simulink model "ofdm_txrx_mimo"  (Simulink 7.1)
settings: Solver=ode45  StartTime=0.0  StopTime=6e3  RelTol=1e-3
PreLoadFcn:
  | ofdm_rx_mimo_init
InitFcn:
  | ofdm_rx_mimo_init
StartFcn:
  | ofdm_rx_mimo_init
values from neighbouring files (symbols this model uses):
  BER_RAM_init_size = 2048    [ofdm_tx_mimo_init.m]
  CPLength = 16    [ofdm_tx_mimo_init.m]
  CRCPolynomial = hex2dec('04c11db7')    [ofdm_tx_mimo_init.m]
  freqOffset_track_filtCoef_i = hex2dec('2500')/2^32    [ofdm_rx_mimo_init.m]
  freqOffset_track_filtCoef_p = hex2dec('d0000')/2^32    [ofdm_rx_mimo_init.m]
  hi = zeros(1,length(cci))    [ofdm_rx_mimo_init.m]
  hr = zeros(1,length(ccr))    [ofdm_rx_mimo_init.m]
  max_CP_length = 16    [ofdm_tx_mimo_init.m]
  max_num_baseRateSymbols = 31    [ofdm_tx_mimo_init.m]
  max_num_subcarriers = 64    [ofdm_tx_mimo_init.m]
  max_num_trainingSymbols = 15    [ofdm_tx_mimo_init.m]
  max_numBytes = 16384    [ofdm_tx_mimo_init.m]
  max_OFDM_symbols = 2047    [ofdm_tx_mimo_init.m]
  modConstellation_qam16 = .75*[1 3 -1 -3]./3    [ofdm_tx_mimo_init.m]
  modConstellation_qam256 = 0.9375*[3 1 5 7 11 9 13 15 -3 -1 -5 -7 -11 -9 -13 -15]./15    [ofdm_tx_mimo_init.m]
  modConstellation_qam64 = 0.875*[3 1 5 7 -3 -1 -5 -7]./7    [ofdm_tx_mimo_init.m]
  modConstellation_qpsk = [1 -1]./sqrt(2)    [ofdm_tx_mimo_init.m]
  numBaseRateSymbols = 2    [ofdm_tx_mimo_init.m]
  numSubcarriers = 64    [ofdm_tx_mimo_init.m]
  numTrainingSymbols = 2    [ofdm_tx_mimo_init.m]
  pilot1_indicies = 7 + ( (64-7) * 2^16)    (= 3735559)    [ofdm_tx_mimo_init.m]
  pilot2_indicies = 21 + ( (64-21) * 2^16)    (= 2818069)    [ofdm_tx_mimo_init.m]
  pilotValue_neg = hex2dec('8000') + (2^16 * 0)    [ofdm_tx_mimo_init.m]
  pilotValue_pos = hex2dec('7FFF') + (2^16 * 0)    [ofdm_tx_mimo_init.m]
  pkt_crossCorr_thresh = 0.7    [ofdm_rx_mimo_init.m]
  pkt_energy_thresh = 0    [ofdm_rx_mimo_init.m]
  pkt_numPayloadBytes = 1400+42-6    (= 1436)    [ofdm_tx_mimo_init.m]
  pktByteNums = numHeaderBytes +  				byteIndex_numPayloadBytes(1)*2^8 +  				byteIndex_numPayloadBytes(2)*2^16 +  				byteIndex_simpleDynModMasks * 2^24    [ofdm_rx_mimo_init.m]
  PktDet_Delay = 26 + Symbol_Timing_Offset*2^7    (= 1050)    [ofdm_rx_mimo_init.m]
  preamble = 6*[0 shortsyms_10 longsyms_2]    [ofdm_tx_mimo_init.m]
  preamble_I = real(preamble)    [ofdm_tx_mimo_init.m]
  preamble_Q = imag(preamble)    [ofdm_tx_mimo_init.m]
  RAM_init_size = max_numBytes    (= 16384)    [ofdm_tx_mimo_init.m]
  reg_Interrupt_PktBuf_Control = reg_InterruptControl +  	1 * 2^16 +  	0 * 2^24    (= 65656)    [ofdm_rx_mimo_init.m]
  rx_controlBits = 1 * 2  		 + 1 * 4  		 + 0 * 8  		 + 1 * 16 * rx_SISO_Mode  		 + 1 * 32  		 + 1 * 64  		 + 0 * 128  		 + 1 * 256  		 + 0 * 512  		 + 1 * 1024  		 + 0 * 2048  		 + 1 * 4096  		 + 1 * 8192  		 + 0 * 16384  		 + 1 * 65536  		 + 1 * 131072  		 + 0 * 262144  		 + 1 * 524288    [ofdm_rx_mimo_init.m]
  Rx_PhaseNoiseTrack_K = 0.6094    [ofdm_rx_mimo_init.m]
  rx_postEq_scaling = round(rxScaling*2^11) * (1 + 2^16)    [ofdm_rx_mimo_init.m]
  RxDataScrambling_Seq = [40 198 78 63 82 173 102 245 48 111 172 115 147 230 216 93 72 65 62 2 205 242 122 90 128 83 105 97 73 10 5 252]    [ofdm_tx_mimo_init.m]
  simOnly_numSamples = length(preamble)+( (numSubcarriers+CPLength)*(numTrainingSymbols + numBaseRateSymbols + numFullRateSymbols/2) )    [ofdm_tx_mimo_init.m]
  train = [train train]    [ofdm_tx_mimo_init.m]
  tx_controlBits = (antB_preambleShift * 2^4) + (2^0 * tx_SISO_Mode) + 4*0 + 8*1    (= 73)    [ofdm_tx_mimo_init.m]
  TxDataScrambling_Seq = [40 198 78 63 82 173 102 245 48 111 172 115 147 230 216 93 72 65 62 2 205 242 122 90 128 83 105 97 73 10 5 252]    [ofdm_tx_mimo_init.m]
  txReg_symbolCounts = (2^16 * numFullRateSymbols) + (2^8 *numBaseRateSymbols ) + numTrainingSymbols    [ofdm_tx_mimo_init.m]
  TxRx_FFTScaling = bin2dec('010111') + (bin2dec('000101') * 2^6)    [ofdm_tx_mimo_init.m]
undefined symbols (not defined in the file or in its folder): AntA, AntB, BaseRate, CRC_table_gen, gcbh, intfilt, pktTiming_count_payloadStart, pktTiming_symbol_length, xlBlockLoadCallback, xlGetSimulinkPeriod
libraries: xbsIndex_r4 x4212, dspmlti4 x4, dspsigops x4, commchan3 x2, simulink x2, commrflib2 x2, dspsrcs4 x1
(part 1 of 43)
settings Model: Creator=Administrator  LastModifiedBy=Administrator

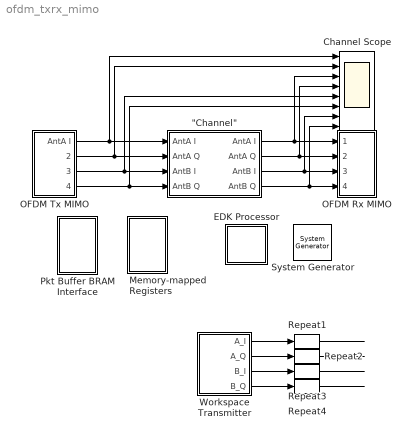
== system ofdm_txrx_mimo ==
  " System Generator" : xbsIndex_r4/ System Generator  Tag=genX  SystemSampleTime=-1  xilinxfamily=virtex2p  part=xc2vp70  speed=-6  package=ff1517  synthesis_tool=XST  clock_wrapper=Clock Enables  directory=./OFDM_txrx_v03_newerTxScaling  testbench=off  simulink_period=1  sysclk_period=10  dcm_input_clock_period=100  incr_netlist=off  trim_vbits=Everywhere in SubSystem  dbl_ovrd=According to Block Masks  core_generation=According to Block Masks  run_coregen=off  deprecated_control=off  eval_field=0  block_type=sysgen
      settings: block_version=8.2.02
  "\"Channel\"" : Subsystem  mask{h=[1 0; 0 1] * 0.3, freqOffset=1e-4, awgnSNR=500, phNoise=-5000}
      mask prompts: h "2x2 Channel Matrix"; freqOffset "Freq Offset (phase inc [0,1])"; awgnSNR "SNR (dB)"; phNoise "Phase Noise"
      mask help: This block implements a simple static 2x2 channel. The one parameter sets the 2x2 channel matrix. h=[1 0;0 1] would be perfectly orthogonal channels. The norm of the matrix should be 1 to avoid overflow.
  Channel Scope : Scope  NumInputPorts=8
      settings: TimeRange=3000  YMin=-1~-1~-0.3~-0.3~-1~-1~-1~-1  YMax=0.75~0.75~0.2~0.2~1~1~1~1  SaveName=ScopeData49
  EDK Processor : Subsystem  LoadFcn=xlBlockLoadCallback(gcbh);  mask(Xilinx EDK Processor Block){mode=EDK pcore generation, xmp=, MemVisToProc=<qt bgcolor="#FFFFFF"><div><img src="C:\Xilinx\10.1\DSP_Tools\common\bin\..\..\sysgen\data\images\registerplus.gif"> &lt;&lt;Rx_BER_Errors&gt;&gt;<br></div><div><img src="C:\Xilinx\10.1\DSP_Tools\common\bin\..\..\sysgen\data\images\registerplus.gif"> &lt;&lt;Rx_BER_TotalBits&gt;&gt;<br></div><div><img src="C:\Xilinx\10.1\DSP_Tools\common\bin\..\..\sysgen\data\images\registerplus.gif"> &lt;&lt;Rx_Constellation_Scaling_w&gt;&gt;<br></div><div><img src="C:\Xilinx\10.1\DSP_Tools\common\bin\..\..\sysgen\data\images\registerplus.gif"> &lt;&lt;Rx_ControlBits_r&gt;&gt;<br></div><div><img src="C:\Xilinx\10.1\DSP_Tools\common\bin\..\..\sysgen\data\images\registerplus.gif"> &lt;&lt;Rx_ControlBits_w&gt;&gt;<br></div><div><img src="C:\Xilinx\10.1\DSP_Tools\common\bin\..\..\sysgen\data\images\registerplus.gif"> &lt;&lt;Rx_EVM_w&gt;&gt;<br></div><div><img src="C:\Xilinx\10.1\DSP_Tools\common\bin\..\..\sysgen\data\images\registerplus.gif"> &lt;&lt;Rx_FreqOffFilt_B_KI_w&gt;&gt;<br></div><div><img src="C:\Xilinx\10.1\DSP_Tools\common\bin\..\..\sysgen\data\images\registerplus.gif"> &lt;&lt;Rx_FreqOffFilt_B_KP_w&gt;&gt;<br></div><div><img src="C:\Xilinx\10.1\DSP_Tools\common\bin\..\..\sysgen\data\images\registerplus.gif"> &lt;&lt;Rx_Gains&gt;&gt;<br></div><div><img src="C:\Xilinx\10.1\DSP_Tools\common\bin\..\..\sysgen\data\images\registerplus.gif"> &lt;&lt;Rx_OFDM_SymbolCounts_r&gt;&gt;<br></div><div><img src="C:\Xilinx\10.1\DSP_Tools\common\bin\..\..\sysgen\data\images\registerplus.gif"> &lt;&lt;Rx_OFDM_SymbolCounts_w&gt;&gt;<br></div><div><img src="C:\Xilinx\10.1\DSP_Tools\common\bin\..\..\sysgen\data\images\registerplus.gif"> &lt;&lt;Rx_PhaseNoiseTrack_K_w&gt;&gt;<br></div><div><img src="C:\Xilinx\10.1\DSP_Tools\common\bin\..\..\sysgen\data\images\registerplus.gif"> &lt;&lt;Rx_PktDet_Delay_r&gt;&gt;<br></div><div><img src="C:\Xilinx\10.1\DSP_Tools\common\bin\..\..\sysgen\data\images\registerplus.gif"> &lt;&lt;Rx_PktDet_Delay_w&gt;&gt;<br></div><div><img src="C:\Xilinx\10.1\DSP_Tools\common\bin\..\..\sysgen\data\images\registerplus.gif"> &lt;&lt;Rx_PktDet_LongCorr_Params_r&gt;&gt;<br></div><div><img src="C:\Xilinx\10.1\DSP_Tools\common\bin\..\..\sysgen\data\images\registerplus.gif"> &lt;&lt;Rx_PktDet_LongCorr_Params_w&gt;&gt;<br></div><div><img src="C:\Xilinx\10.1\DSP_Tools\common\bin\..\..\sysgen\data\images\registerplus.gif"> &lt;&lt;Rx_collision&gt;&gt;<br></div><div><img src="C:\Xilinx\10.1\DSP_Tools\common\bin\..\..\sysgen\data\images\registerplus.gif"> &lt;&lt;Rx_pktByteNums_r&gt;&gt;<br></div><div><img src="C:\Xilinx\10.1\DSP_Tools\common\bin\..\..\sysgen\data\images\registerplus.gif"> &lt;&lt;Rx_pktByteNums_w&gt;&gt;<br></div><div><img src="C:\Xilinx\10.1\DSP_Tools\common\bin\..\..\sysgen\data\images\registerplus.gif"> &lt;&lt;Rx_pktDet_Tresholds_w&gt;&gt;<br></div><div><img src="C:\Xilinx\10.1\DSP_Tools\common\bin\..\..\sysgen\data\images\registerplus.gif"> &lt;&lt;Rx_pktDone_interruptStatus&gt;&gt;<br></div><div><img src="C:\Xilinx\10.1\DSP_Tools\common\bin\..\..\sysgen\data\images\registerplus.gif"> &lt;&lt;TxRx_FFT_Scaling_w&gt;&gt;<br></div><div><img src="C:\Xilinx\10.1\DSP_Tools\common\bin\..\..\sysgen\data\images\registerplus.gif"> &lt;&lt;TxRx_Interrupt_PktBuf_Ctrl_r&gt;&gt;<br></div><div><img src="C:\Xilinx\10.1\DSP_Tools\common\bin\..\..\sysgen\data\images\registerplus.gif"> &lt;&lt;TxRx_Interrupt_PktBuf_Ctrl_w&gt;&gt;<br></div><div><img src="C:\Xilinx\10.1\DSP_Tools\common\bin\..\..\sysgen\data\images\registerplus.gif"> &lt;&lt;Tx_ControlBits_r&gt;&gt;<br></div><div><img src="C:\Xilinx\10.1\DSP_Tools\common\bin\..\..\sysgen\data\images\registerplus.gif"> &lt;&lt;Tx_ControlBits_w&gt;&gt;<br></div><div><img src="C:\Xilinx\10.1\DSP_Tools\common\bin\..\..\sysgen\data\images\registerplus.gif"> &lt;&lt;Tx_OFDM_SymCounts_r&gt;&gt;<br></div><div><img src="C:\Xilinx\10.1\DSP_Tools\common\bin\..\..\sysgen\data\images\registerplus.gif"> &lt;&lt;Tx_OFDM_SymCounts_w&gt;&gt;<br></div><div><img src="C:\Xilinx\10.1\DSP_Tools\common\bin\..\..\sysgen\data\images\registerplus.gif"> &lt;&lt;Tx_Pilots_Index1_w&gt;&gt;<br></div><div><img src="C:\Xilinx\10.1\DSP_Tools\common\bin\..\..\sysgen\data\images\registerplus.gif"> &lt;&lt;Tx_Pilots_Index2_w&gt;&gt;<br></div><div><img src="C:\Xilinx\10.1\DSP_Tools\common\bin\..\..\sysgen\data\images\registerplus.gif"> &lt;&lt;Tx_Pilots_Value1_w&gt;&gt;<br></div><div><img src="C:\Xilinx\10.1\DSP_Tools\common\bin\..\..\sysgen\data\images\registerplus.gif"> &lt;&lt;Tx_Pilots_Value2_w&gt;&gt;<br></div><div><img src="C:\Xilinx\10.1\DSP_Tools\common\bin\..\..\sysgen\data\images\registerplus.gif"> &lt;&lt;Tx_PktRunning&gt;&gt;<br></div><div><img src="C:\Xilinx\10.1\DSP_Tools\common\bin\..\..\sysgen\data\images\registerplus.gif"> &lt;&lt;Tx_PreambleMasking_w&gt;&gt;<br></div><div><img src="C:\Xilinx\10.1\DSP_Tools\common\bin\..\..\sysgen\data\images\registerplus.gif"> &lt;&lt;Tx_Scaling_w&gt;&gt;<br></div><div><img src="C:\Xilinx\10.1\DSP_Tools\common\bin\..\..\sysgen\data\images\registerplus.gif"> &lt;&lt;Tx_Start_Reset_Control_r&gt;&gt;<br></div><div><img src="C:\Xilinx\10.1\DSP_Tools\common\bin\..\..\sysgen\data\images\registerplus.gif"> &lt;&lt;Tx_Start_Reset_Control_w&gt;&gt;<br></div><div><img src="C:\Xilinx\10.1\DSP_Tools\common\bin\..\..\sysgen\data\images\registerplus.gif"> &lt;&lt;midPacketRSSI&gt;&gt;<br></div><div><img src="C:\Xilinx\10.1\DSP_Tools\common\bin\..\..\sysgen\data\images\registerplus.gif"> &lt;&lt;pktDet_controlBits_r&gt;&gt;<br></div><div><img src="C:\Xilinx\10.1\DSP_Tools\common\bin\..\..\sysgen\data\images\registerplus.gif"> &lt;&lt;pktDet_controlBits_w&gt;&gt;<br></div><div><img src="C:\Xilinx\10.1\DSP_Tools\common\bin\..\..\sysgen\data\images\registerplus.gif"> &lt;&lt;pktDet_durations_r&gt;&gt;<br></div><div><img src="C:\Xilinx\10.1\DSP_Tools\common\bin\..\..\sysgen\data\images\registerplus.gif"> &lt;&lt;pktDet_durations_w&gt;&gt;<br></div><div><img src="C:\Xilinx\10.1\DSP_Tools\common\bin\..\..\sysgen\data\images\registerplus.gif"> &lt;&lt;pktDet_status&gt;&gt;<br></div><div><img src="C:\Xilinx\10.1\DSP_Tools\common\bin\..\..\sysgen\data\images\registerplus.gif"> &lt;&lt;pktDet_thresholds_r&gt;&gt;<br></div><div><img src="C:\Xilinx\10.1\DSP_Tools\common\bin\..\..\sysgen\data\images\registerplus.gif"> &lt;&lt;pktDet_thresholds_w&gt;&gt;<br></div><div><img src="C:\Xilinx\10.1\DSP_Tools\common\bin\..\..\sysgen\data\images\memoryplus.gif"> &lt;&lt;RxModulation&gt;&gt;<br></div><div><img src="C:\Xilinx\10.1\DSP_Tools\common\bin\..\..\sysgen\data\images\memoryplus.gif"> &lt;&lt;TxModulation&gt;&gt;<br></div></qt>, AvailableMemories=<empty>, portInterfaceTable={'exposed'=>[],'portdir'=>[],'portname'=>[],'shortname'=>[]}, bus_type_sgadvanced=, bus_type=PLB, baseaddr=0x80000000, baseaddr_lock_sgadvanced=, baseaddr_lock=off, dual_clock_sgadvanced=, dual_clock=off, reg_readback_sgadvanced=, reg_readback=off, ucf_file=, inheritDeviceType_sgadvanced=, inheritDeviceType=off, clock_name=plb, internalPortList={}, resetPolarity=0, memxtable=|, procinfo={'xmliface'=>'Xilinx//microblaze//iface.xml'}, memmapdirty=off, blockname=, xpsintstyle=default, has_advanced_control=0, sggui_pos=20,20,383,441, block_type=edkprocessor, block_version=2.5, sg_icon_stat=38,36,-1,-1,white,blue,0,07734,right, sg_mask_display=|, sg_list_contents={'table'=>{'AvailableMemories'=>'popup(<empty>)','userSelections'=>{'AvailableMemories'=>'<empty>'}}}, sg_blockgui_xml=}
      mask memxtable=|
      | {'mlist'=>['ofdm_txrx_mimo/Memory-mapped
      | Registers/Rx Registers/Read Only Register1/To Register','ofdm_txrx_mimo/Memory-mapped
      | Registers/Rx Registers/Read Only Register2/To Register','ofdm_txrx_mimo/Memory-mapped
      | Registers/Rx Registers/Write-Only Register1/From Register','ofdm_txrx_mimo/Memory-mapped
      | Registers/Rx Registers/Read-Write Register5/To Register','ofdm_txrx_mimo/Memory-mapped
      | Registers/Rx Registers/Read-Write Register5/From Register','ofdm_txrx_mimo/Memory-mapped
      | Registers/Rx Registers/Write-Only Register5/From Register','ofdm_txrx_mimo/Memory-mapped
      | Registers/Rx Registers/Write-Only Register2/From Register','ofdm_txrx_mimo/Memory-mapped
      | Registers/Rx Registers/Write-Only Register3/From Register','ofdm_txrx_mimo/Memory-mapped
      | Registers/Rx Registers/Read Only Register/To Register','ofdm_txrx_mimo/Memory-mapped
      | Registers/Rx Registers/Read-Write Register4/To Register','ofdm_txrx_mimo/Memory-mapped
      | Registers/Rx Registers/Read-Write Register4/From Register','ofdm_txrx_mimo/Memory-mapped
      | Registers/Rx Registers/Write-Only Register4/From Register','ofdm_txrx_mimo/Memory-mapped
      | Registers/Rx Registers/Read-Write Register3/To Register','ofdm_txrx_mimo/Memory-mapped
      | Registers/Rx Registers/Read-Write Register3/From Register','ofdm_txrx_mimo/Memory-mapped
      | Registers/Rx Registers/Read-Write Register1/To Register','ofdm_txrx_mimo/Memory-mapped
      | Registers/Rx Registers/Read-Write Register1/From Register','ofdm_txrx_mimo/OFDM Rx MIMO/Equalizer & Packetizer/EVM/To Register','ofdm_txrx_mimo/Memory-mapped
      | Registers/Rx Registers/Read-Write Register2/To Register','ofdm_txrx_mimo/Memory-mapped
      | Registers/Rx Registers/Read-Write Register2/From Register','ofdm_txrx_mimo/Memory-mapped
      | Registers/Rx Registers/Write-Only Register/From Register','ofdm_txrx_mimo/Memory-mapped
      | Registers/Rx Registers/Read Only Register3/To Register','ofdm_txrx_mimo/Memory-mapped
      | Registers/TxRx Registers/Write-Only Register/From Register','ofdm_txrx_mimo/Memory-mapped
      | Registers/TxRx Registers/Read-Write Register/To Register','ofdm_txrx_mimo/Memory-mapped
      | Registers/TxRx Registers/Read-Write Register/From Register','ofdm_txrx_mimo/Memory-mapped
      | Registers/Tx Registers/Read-Write Register1/To Register','ofdm_txrx_mimo/Memory-mapped
      | Registers/Tx Registers/Read-Write Register1/From Register','ofdm_txrx_mimo/Memory-mapped
      | Registers/Tx Registers/Read-Write Register2/To Register','ofdm_txrx_mimo/Memory-mapped
      | Registers/Tx Registers/Read-Write Register2/From Register','ofdm_txrx_mimo/Memory-mapped
      | Registers/Tx Registers/Write-Only Register2/From Register','ofdm_txrx_mimo/Memory-mapped
      | Registers/Tx Registers/Write-Only Register3/From Register','ofdm_txrx_mimo/Memory-mapped
      | Registers/Tx Registers/Write-Only Register4/From Register','ofdm_txrx_mimo/Memory-mapped
      | Registers/Tx Registers/Write-Only Register5/From Register','ofdm_txrx_mimo/Memory-mapped
      | Registers/Tx Registers/Read Only Register/To Register','ofdm_txrx_mimo/Memory-mapped
      | Registers/Tx Registers/Write-Only Register/From Register','ofdm_txrx_mimo/Memory-mapped
      | Registers/Tx Registers/Write-Only Register1/From Register','ofdm_txrx_mimo/Memory-mapped
      | Registers/Tx Registers/Read-Write Register/To Register','ofdm_txrx_mimo/Memory-mapped
      | Registers/Tx Registers/Read-Write Register/From Register','ofdm_txrx_mimo/OFDM Rx MIMO/Packet_Detection/RSSI-based PktDet/Mid-Packet
      | RSSI Register/To Register2','ofdm_txrx_mimo/OFDM Rx MIMO/Packet_Detection/RSSI-based PktDet/Pkt Detector Registers/Read-Write Register1/To Register','ofdm_txrx_mimo/OFDM Rx MIMO/Packet_Detection/RSSI-based PktDet/Pkt Detector Registers/Read-Write Register1/From Register','ofdm_txrx_mimo/OFDM Rx MIMO/Packet_Detection/RSSI-based PktDet/Pkt Detector Registers/Read-Write Register2/To Register','ofdm_txrx_mimo/OFDM Rx MIMO/Packet_Detection/RSSI-based PktDet/Pkt Detector Registers/Read-Write Register2/From Register','ofdm_txrx_mimo/OFDM Rx MIMO/Packet_Detection/RSSI-based PktDet/Pkt Detector Registers/To Register','ofdm_txrx_mimo/OFDM Rx MIMO/Packet_Detection/RSSI-based PktDet/Pkt Detector Registers/Read-Write Register5/To Register','ofdm_txrx_mimo/OFDM Rx MIMO/Packet_Detection/RSSI-based PktDet/Pkt Detector Registers/Read-Write Register5/From Register','ofdm_txrx_mimo/OFDM Rx MIMO/Equalizer & Packetizer/Packet_Constructor/Modulation RAM/Non-shared (read-only)
      | Modulation Masks/Shared Memory','ofdm_txrx_mimo/OFDM Tx MIMO/Training_Data/FlexibleMod/DataModulation/control/Tx Modulation/Non-shared (read-only)
      | Modulation Masks/Shared Memory'],'mlname'=>['\'Rx_BER_Errors\'','\'Rx_BER_TotalBits\'','\'Rx_Constellation_Scaling_w\'','\'Rx_ControlBits_r\'','\'Rx_ControlBits_w\'','\'Rx_EVM_w\'','\'Rx_FreqOffFilt_B_KI_w\'','\'Rx_FreqOffFilt_B_KP_w\'','\'Rx_Gains\'','\'Rx_OFDM_SymbolCounts_r\'','\'Rx_OFDM_SymbolCounts_w\'','\'Rx_PhaseNoiseTrack_K_w\'','\'Rx_PktDet_Delay_r\'','\'Rx_PktDet_Delay_w\'','\'Rx_PktDet_LongCorr_Params_r\'','\'Rx_PktDet_LongCorr_Params_w\'','\'Rx_collision\'','\'Rx_pktByteNums_r\'','\'Rx_pktByteNums_w\'','\'Rx_pktDet_Tresholds_w\'','\'Rx_pktDone_interruptStatus\'','\'TxRx_FFT_Scaling_w\'','\'TxRx_Interrupt_PktBuf_Ctrl_r\'','\'TxRx_Interrupt_PktBuf_Ctrl_w\'','\'Tx_ControlBits_r\'','\'Tx_ControlBits_w\'','\'Tx_OFDM_SymCounts_r\'','\'Tx_OFDM_SymCounts_w\'','\'Tx_Pilots_Index1_w\'','\'Tx_Pilots_Index2_w\'','\'Tx_Pilots_Value1_w\'','\'Tx_Pilots_Value2_w\'','\'Tx_PktRunning\'','\'Tx_PreambleMasking_w\'','\'Tx_Scaling_w\'','\'Tx_Start_Reset_Control_r\'','\'Tx_Start_Reset_Control_w\'','\'midPacketRSSI\'','\'pktDet_controlBits_r\'','\'pktDet_controlBits_w\'','\'pktDet_durations_r\'','\'pktDet_durations_w\'','\'pktDet_status\'','\'pktDet_thresholds_r\'','\'pktDet_thresholds_w\'','\'RxModulation\'','\'TxModulation\''],'mlstate'=>[0.00000000000000000,0.00000000000000000,0.00000000000000000,0.00000000000000000,0.00000000000000000,0.00000000000000000,0.00000000000000000,0.00000000000000000,0.00000000000000000,0.00000000000000000,0.00000000000000000,0.00000000000000000,0.00000000000000000,0.00000000000000000,0.00000000000000000,0.00000000000000000,0.00000000000000000,0.00000000000000000,0.00000000000000000,0.00000000000000000,0.00000000000000000,0.00000000000000000,0.00000000000000000,0.00000000000000000,0.00000000000000000,0.00000000000000000,0.00000000000000000,0.00000000000000000,0.00000000000000000,0.00000000000000000,0.00000000000000000,0.00000000000000000,0.00000000000000000,0.00000000000000000,0.00000000000000000,0.00000000000000000,0.00000000000000000,0.00000000000000000,0.00000000000000000,0.00000000000000000,0.00000000000000000,0.00000000000000000,0.00000000000000000,0.00000000000000000,0.00000000000000000,0.00000000000000000,0.00000000000000000]}
      mask sg_mask_display=|
      | fprintf('','COMMENT: begin icon graphics');
      | patch([0 38 38 0 ],[0 0 36 36 ],[0.77 0.82 0.91]);
      | patch([9 3 11 3 9 19 22 25 35 27 19 13 21 13 19 27 35 25 22 19 9 ],[4 10 18 26 32 32 29 32 32 24 32 26 18 10 4 12 4 4 7 4 4 ],[0.98 0.96 0.92]);
      | plot([0 38 38 0 0 ],[0 0 36 36 0 ]);
      | fprintf('','COMMENT: end icon graphics');
      | fprintf('','COMMENT: begin icon text');
      | fprintf('','COMMENT: end icon text');
      mask prompts: mode "Configure Processor for"; xmp "EDK Project"; MemVisToProc ""; AvailableMemories "Available Memories"; portInterfaceTable ""; bus_type_sgadvanced ""; bus_type "Bus Type"; baseaddr "Base Address"; baseaddr_lock_sgadvanced ""; baseaddr_lock "Lock"; dual_clock_sgadvanced ""; dual_clock "Dual Clocks"; reg_readback_sgadvanced ""; reg_readback "Register Read-Back"; ucf_file "Constraint file"; inheritDeviceType_sgadvanced ""; inheritDeviceType "Inherit Device Type"; clock_name ""; internalPortList ""; resetPolarity ""; memxtable ""; procinfo ""; memmapdirty ""; blockname ""; xpsintstyle ""; has_advanced_control ""; sggui_pos ""; block_type ""; block_version ""; sg_icon_stat ""; sg_mask_display ""; sg_list_contents ""; sg_blockgui_xml ""
      mask help: Xilinx EDK Processor
      mask init:
      | try
      |   tmp_gcb = gcb;
      |   tmp_gcbh = gcbh;
      |   if (strcmp('SysGenIndex',get_param(bdroot(tmp_gcbh),'tag')) && ~isempty(regexp(bdroot(tmp_gcb), '^xbs', 'once')))
      |     return;
      |   end;
      |   xlMungeMaskParams;
      | 
      | block_type='edkprocessor';
      |   serialized_declarations = '{,''block_type''=>''String''}';
      |   xledkprocessor_init();
      |   ptable_ = xlblockprep(get_param(tmp_gcb, 'MaskWSVariables'));
      | catch
      |   global dbgsysgen;
      |   if(~isempty(dbgsysgen) && dbgsysgen)
      |     e = regexprep(lasterr, '\n', '\nError: ');
      |     disp(['Error: While running MaskInit code on block ' tmp_gcb ': ' e]);
      |     error(e);
      |   end
      | end
      settings: CopyFcn=xlProcBlockCopyCallback(gcbh);xlBlockMoveCallback(gcbh);  DeleteFcn=xlDestroyGui(gcbh);  ModelCloseFcn=xlDestroyGui(gcbh);  PreSaveFcn=xlBlockPreSaveCallback(gcbh);  PostSaveFcn=xlBlockPostSaveCallback(gcbh);  DestroyFcn=xlDestroyGui(gcbh);  OpenFcn=bh=gcbh;xlProcBlockCallbacks('populatesharedmemorylistbox',bh);xlOpenGui(bh, 'edkprocessor_gui.xml', @xlProcBlockEnablement, @xlProcBlockAction)  CloseFcn=xlDestroyGui(gcbh);  MoveFcn=xlBlockMoveCallback(gcbh);
      mask Help: eval('');xlDoc('-book','sysgen','-topic','EDK_Processor');
      mask StyleString: popup(EDK pcore generation|HDL netlisting),edit,edit,popup(<empty>),edit,edit,popup(PLB|FSL),edit,edit,checkbox,edit,checkbox,edit,checkbox,edit,edit,checkbox,edit,edit,edit,edit,edit,edit,edit,edit,edit,edit,edit,edit,edit,edit,edit,edit
      mask Display:
      | fprintf('','COMMENT: begin icon graphics');
      | patch([0 38 38 0 ],[0 0 36 36 ],[0.77 0.82 0.91]);
      | patch([9 3 11 3 9 19 22 25 35 27 19 13 21 13 19 27 35 25 22 19 9 ],[4 10 18 26 32 32 29 32 32 24 32 26 18 10 4 12 4 4 7 4 4 ],[0.98 0.96 0.92]);
      | plot([0 38 38 0 0 ],[0 0 36 36 0 ]);
      | fprintf('','COMMENT: end icon graphics');
      | fprintf('','COMMENT: begin icon text');
      | disp('');
      | 
      | fprintf('','COMMENT: end icon text');
  Memory-mapped Registers : Subsystem
  OFDM Rx MIMO : Subsystem
  OFDM Tx MIMO : Subsystem
  Pkt Buffer BRAM Interface : Subsystem
  Repeat{1..4} (4 alike) : dspsigops/Repeat  N=4  ic=0  mode=Maintain input frame size
  Workspace Transmitter : Subsystem
  wires:
  "\"Channel\""/1(" AntA I") -> Channel Scope/3, OFDM Rx MIMO/1(AntA_I)
  "\"Channel\""/2(" AntA Q") -> Channel Scope/4, OFDM Rx MIMO/2(AntA_Q)
  "\"Channel\""/3(" AntB I") -> Channel Scope/7, OFDM Rx MIMO/3(AntB_I)
  "\"Channel\""/4(" AntB Q") -> Channel Scope/8, OFDM Rx MIMO/4(AntB_Q)
  OFDM Tx MIMO/1(AntA I) -> "\"Channel\""/1(AntA I), Channel Scope/1
  OFDM Tx MIMO/2(AntA Q) -> "\"Channel\""/2(AntA Q), Channel Scope/2
  OFDM Tx MIMO/3(AntB I) -> "\"Channel\""/3(AntB I), Channel Scope/5
  OFDM Tx MIMO/4(AntB Q) -> Channel Scope/6, "\"Channel\""/4(AntB Q)
  Workspace Transmitter/2(A_Q) -> Repeat2/1
  Workspace Transmitter/3(B_I) -> Repeat3/1
  Workspace Transmitter/4(B_Q) -> Repeat4/1
  Repeat2/1 -> ?
  Repeat3/1 -> ?
  Repeat4/1 -> ?
  Workspace Transmitter/1(A_I) -> Repeat1/1
  Repeat1/1 -> ?

== system ofdm_txrx_mimo/"Channel" ==
  AntA I : Inport  OutDataType=sfix(16)  OutScaling=2^0
  AntA Q : Inport  Port=2  OutDataType=sfix(16)  OutScaling=2^0
  AntB I : Inport  Port=3  OutDataType=sfix(16)  OutScaling=2^0
  AntB Q : Inport  Port=4  OutDataType=sfix(16)  OutScaling=2^0
  AWGN Channel : commchan3/AWGN Channel  SystemSampleTime=-1  seed=rand  noiseMode="Signal to noise ratio  (SNR)"  EbNodB=10  EsNodB=10  SNRdB=awgnSNR  bitsPerSym=1  Ps=1  Tsym=1  variance=1
  AWGN Channel1 : commchan3/AWGN Channel  SystemSampleTime=-1  seed=rand  noiseMode="Signal to noise ratio  (SNR)"  EbNodB=10  EsNodB=10  SNRdB=awgnSNR  bitsPerSym=1  Ps=1  Tsym=1  variance=1
  Complex to Real-Imag1 : ComplexToRealImag
  Complex to Real-Imag2 : ComplexToRealImag
  FIR Decimation1 : dspmlti4/FIR Decimation  DialogController=dspdialog.FIRDecimation  DialogControllerArgs=DataTag1  h=firpm(31,[0 .25 .3 1],[1 1 0 0])  D=4  filtStruct=Direct form  framing=Maintain input frame size  outputBufInitCond=0  roundingMode=Floor  overflowMode=off  FilterSource=Specify via dialog  FilterObject=Hm_firdecim
  FIR Decimation2 : dspmlti4/FIR Decimation  DialogController=dspdialog.FIRDecimation  DialogControllerArgs=DataTag2  h=firpm(31,[0 .25 .3 1],[1 1 0 0])  D=4  filtStruct=Direct form  framing=Maintain input frame size  outputBufInitCond=0  roundingMode=Floor  overflowMode=off  FilterSource=Specify via dialog  FilterObject=Hm_firdecim
  FIR Decimation3 : dspmlti4/FIR Decimation  DialogController=dspdialog.FIRDecimation  DialogControllerArgs=DataTag3  h=firpm(31,[0 .25 .3 1],[1 1 0 0])  D=4  filtStruct=Direct form  framing=Maintain input frame size  outputBufInitCond=0  roundingMode=Floor  overflowMode=off  FilterSource=Specify via dialog  FilterObject=Hm_firdecim
  FIR Decimation4 : dspmlti4/FIR Decimation  DialogController=dspdialog.FIRDecimation  DialogControllerArgs=DataTag4  h=firpm(31,[0 .25 .3 1],[1 1 0 0])  D=4  filtStruct=Direct form  framing=Maintain input frame size  outputBufInitCond=0  roundingMode=Floor  overflowMode=off  FilterSource=Specify via dialog  FilterObject=Hm_firdecim
  Freq Offset : Subsystem
  Gain : Gain  ParameterDataType=sfix(16)  ParameterScaling=2^0  OutDataType=sfix(16)  OutScaling=2^0
  Gain1 : Gain  ParameterDataType=sfix(16)  ParameterScaling=2^0  OutDataType=sfix(16)  OutScaling=2^0
  Integer Delay : simulink/Discrete/Integer Delay  vinit=0.0  samptime=-1  NumDelays=4
  Integer Delay1 : simulink/Discrete/Integer Delay  vinit=0.0  samptime=-1  NumDelays=4
  "MIMO \"Channel\"" : Subsystem
  Phase Noise : commrflib2/Phase Noise  SystemSampleTime=-1  PhNs=phNoise  Fo=100  seed=rand
      settings: AttributesFormatString=\n
  Phase Noise1 : commrflib2/Phase Noise  SystemSampleTime=-1  PhNs=phNoise  Fo=100  seed=rand
      settings: AttributesFormatString=\n
  Real-Imag to Complex : RealImagToComplex
  Real-Imag to Complex1 : RealImagToComplex
  Zero-Order Hold : ZeroOrderHold
  Zero-Order Hold1 : ZeroOrderHold
  " AntA I" : Outport  OutDataType=sfix(16)  OutScaling=2^0
  " AntA Q" : Outport  Port=2  OutDataType=sfix(16)  OutScaling=2^0
  " AntB I" : Outport  Port=3  OutDataType=sfix(16)  OutScaling=2^0
  " AntB Q" : Outport  Port=4  OutDataType=sfix(16)  OutScaling=2^0
  wires:
  AntA I/1 -> Real-Imag to Complex/1
  AntA Q/1 -> Real-Imag to Complex/2
  AntB I/1 -> Real-Imag to Complex1/1
  AntB Q/1 -> Real-Imag to Complex1/2
  Complex to Real-Imag1/1 -> FIR Decimation3/1
  Complex to Real-Imag1/2 -> FIR Decimation4/1
  Complex to Real-Imag2/1 -> FIR Decimation2/1
  Complex to Real-Imag2/2 -> FIR Decimation1/1
  "MIMO \"Channel\""/1(Out1) -> Freq Offset/1(In1)
  Freq Offset/1(Out1) -> Gain/1
  "MIMO \"Channel\""/2(Out2) -> Freq Offset/2(In2)
  Freq Offset/2(Out2) -> Gain1/1
  Gain/1 -> Integer Delay/1
  AWGN Channel/1 -> Complex to Real-Imag1/1
  Gain1/1 -> Integer Delay1/1
  AWGN Channel1/1 -> Complex to Real-Imag2/1
  Real-Imag to Complex/1 -> Zero-Order Hold/1
  Real-Imag to Complex1/1 -> Zero-Order Hold1/1
  Phase Noise/1 -> AWGN Channel/1
  Phase Noise1/1 -> AWGN Channel1/1
  FIR Decimation3/1 -> " AntA I"/1
  FIR Decimation4/1 -> " AntA Q"/1
  FIR Decimation2/1 -> " AntB I"/1
  FIR Decimation1/1 -> " AntB Q"/1
  Integer Delay1/1 -> Phase Noise1/1
  Integer Delay/1 -> Phase Noise/1
  Zero-Order Hold/1 -> "MIMO \"Channel\""/1(In1)
  Zero-Order Hold1/1 -> "MIMO \"Channel\""/2(In2)
